simulink model "ofdm_txrx_supermimo_coded"  (Simulink 7.7)
settings: Solver=VariableStepDiscrete  SolverMode=SingleTasking  StartTime=0.0  StopTime=2*simOnly_simLength  RelTol=1e-3
PostLoadFcn:
  | ofdm_rx_supermimo_init
InitFcn:
  | ofdm_rx_supermimo_init
StartFcn:
  | ofdm_rx_supermimo_init
values from neighbouring files (symbols this model uses):
  ActionID_AFTransmit = 31    [ofdm_tx_supermimo_init.m]
  ActionID_Disabled = 0    [ofdm_tx_supermimo_init.m]
  ActionID_SetFlagA = 62    [ofdm_tx_supermimo_init.m]
  ActionID_SetFlagB = 61    [ofdm_tx_supermimo_init.m]
  BER_RAM_init_size = 2048    [ofdm_tx_supermimo_init.m]
  channel_h = [1 0; 1 0] * 0.3    [ofdm_tx_supermimo_init.m]
  CPLength = 16    [ofdm_tx_supermimo_init.m]
  longCorr_coef_i = [-4*real(LTS) 0]    [ofdm_rx_supermimo_init.m]
  longCorr_coef_q = [4*imag(LTS) 0]    [ofdm_rx_supermimo_init.m]
  max_CP_length = 16    [ofdm_tx_supermimo_init.m]
  max_num_baseRateSymbols = 31    [ofdm_tx_supermimo_init.m]
  max_num_subcarriers = 64    [ofdm_tx_supermimo_init.m]
  max_num_trainingSymbols = 15    [ofdm_tx_supermimo_init.m]
  max_numBytes = 16384    [ofdm_tx_supermimo_init.m]
  max_OFDM_symbols = 1023    [ofdm_tx_supermimo_init.m]
  modConstellation_qam16 = .75*[1 3 -1 -3]./3    [ofdm_tx_supermimo_init.m]
  modConstellation_qam64 = 0.875*[3 1 5 7 -3 -1 -5 -7]./7    [ofdm_tx_supermimo_init.m]
  modConstellation_qpsk = [1 -1]./sqrt(2)    [ofdm_tx_supermimo_init.m]
  numBaseRateSymbols = numBaseRateSymbols *2    [ofdm_tx_supermimo_init.m]
  numSubcarriers = 64    [ofdm_tx_supermimo_init.m]
  numTrainingSymbols = 2    [ofdm_tx_supermimo_init.m]
  pktTiming_count_payloadStart = pktTiming_count_lsStart + pktTiming_ls_num*pktTiming_ls_length    [ofdm_rx_supermimo_init.m]
  preamble = preamble_scale*[shortsyms_10 longsyms_2]    [ofdm_tx_supermimo_init.m]
  preamble_ROM = preamble_I_ROM + preamble_Q_ROM*2^16    [ofdm_tx_supermimo_init.m]
  RAM_init_size = max_numBytes    (= 16384)    [ofdm_tx_supermimo_init.m]
  RxDataScrambling_Seq = [40 198 78 63 82 173 102 245 48 111 172 115 147 230 216 93 72 65 62 2 205 242 122 90 128 83 105 97 73 10 5 252]    [ofdm_tx_supermimo_init.m]
  shortSymbol_time = shortSymbol_time(1:16).'    [ofdm_tx_supermimo_init.m]
  simOnly_numSamples = length(preamble)+( (numSubcarriers+CPLength)*(numTrainingSymbols + numBaseRateSymbols + numFullRateSymbols/2) )    [ofdm_tx_supermimo_init.m]
  train = [train train]    [ofdm_tx_supermimo_init.m]
  TxDataScrambling_Seq = [40 198 78 63 82 173 102 245 48 111 172 115 147 230 216 93 72 65 62 2 205 242 122 90 128 83 105 97 73 10 5 252]    [ofdm_tx_supermimo_init.m]
undefined symbols (not defined in the file or in its folder): AntA, AntB, BaseRate, Down, gcbh, numBuffs, numBytes, Up, xlBlockLoadCallback, xlGetNormalizedPeriod, xlipgetpartsetting
libraries: xbsIndex_r4 x5430
(part 34 of 49)

== system ofdm_txrx_supermimo_coded/OFDM Tx MIMO/Training_Data/FlexibleMod/PktBuffer_CRC1/Header Decoding1 ==
  TxByteAddr : Inport
  TxByte : Inport  Port=2
  TxByteAddrVin : Inport  Port=3
  Rst : Inport  Port=4
  4LSB : xbsIndex_r4/Slice  nbits=4  boolean_output=off  mode=Lower Bit Location + Width  bit1=0  base1=MSB of Input  bit0=0  base0=LSB of Input  dbl_ovrd=off  block_type=slice
      settings: infoedit="Extracts a given range of bits from each input sample and presents it at the output.  The output type is ordinarily unsigned with binary point at zero, but can be Boolean when the slice is one bit wide.<P><P>Hardware notes: In hardware this block costs nothing."  block_version=VER_STRING_GOES_HERE
  4MSB : xbsIndex_r4/Slice  nbits=4  boolean_output=off  mode=Upper Bit Location + Width  bit1=0  base1=MSB of Input  bit0=0  base0=LSB of Input  dbl_ovrd=off  block_type=slice
      settings: infoedit="Extracts a given range of bits from each input sample and presents it at the output.  The output type is ordinarily unsigned with binary point at zero, but can be Boolean when the slice is one bit wide.<P><P>Hardware notes: In hardware this block costs nothing."  block_version=VER_STRING_GOES_HERE
  8LSB : xbsIndex_r4/Slice  nbits=8  boolean_output=off  mode=Lower Bit Location + Width  bit1=0  base1=MSB of Input  bit0=0  base0=LSB of Input  dbl_ovrd=off  block_type=slice
      settings: infoedit="Extracts a given range of bits from each input sample and presents it at the output.  The output type is ordinarily unsigned with binary point at zero, but can be Boolean when the slice is one bit wide.<P><P>Hardware notes: In hardware this block costs nothing."  block_version=VER_STRING_GOES_HERE
  8LSB+8 : xbsIndex_r4/Slice  nbits=8  boolean_output=off  mode=Lower Bit Location + Width  bit1=0  base1=MSB of Input  bit0=8  base0=LSB of Input  dbl_ovrd=off  block_type=slice
      settings: infoedit="Extracts a given range of bits from each input sample and presents it at the output.  The output type is ordinarily unsigned with binary point at zero, but can be Boolean when the slice is one bit wide.<P><P>Hardware notes: In hardware this block costs nothing."  block_version=VER_STRING_GOES_HERE
  8MSB : xbsIndex_r4/Slice  nbits=8  boolean_output=off  mode=Upper Bit Location + Width  bit1=0  base1=MSB of Input  bit0=0  base0=LSB of Input  dbl_ovrd=off  block_type=slice
      settings: infoedit="Extracts a given range of bits from each input sample and presents it at the output.  The output type is ordinarily unsigned with binary point at zero, but can be Boolean when the slice is one bit wide.<P><P>Hardware notes: In hardware this block costs nothing."  block_version=VER_STRING_GOES_HERE
  Assert1 : xbsIndex_r4/Assert  assert_type=off  type_source=Explicitly  gui_display_data_type=Fixed-point  arith_type="Signed  (2's comp)"  n_bits=16  bin_pt=14  preci_type=Single  exp_width=8  frac_width=24  assert_rate=on  rate_source=Explicitly  period=2  output_port=on  block_type=assert
      settings: infoedit=Asserts a user-defined sample rate and/or type on a signal.<P><P>Hardware notes: In hardware this block costs nothing.  block_version=VER_STRING_GOES_HERE
  Assert2 : xbsIndex_r4/Assert  assert_type=off  type_source=Explicitly  gui_display_data_type=Fixed-point  arith_type="Signed  (2's comp)"  n_bits=16  bin_pt=14  preci_type=Single  exp_width=8  frac_width=24  assert_rate=on  rate_source=Explicitly  period=2  output_port=on  block_type=assert
      settings: infoedit=Asserts a user-defined sample rate and/or type on a signal.<P><P>Hardware notes: In hardware this block costs nothing.  block_version=VER_STRING_GOES_HERE
  Logical1 : xbsIndex_r4/Logical  logical_function=AND  inputs=2  en=off  latency=0  precision=Full  arith_type=Unsigned  n_bits=8  bin_pt=2  align_bp=on  dbl_ovrd=off  block_type=logical
      settings: xl_area=[1 0 0 1 0 0 0]  block_version=VER_STRING_GOES_HERE
  Logical2 : xbsIndex_r4/Logical  logical_function=AND  inputs=2  en=off  latency=0  precision=Full  arith_type=Unsigned  n_bits=8  bin_pt=2  align_bp=on  dbl_ovrd=off  block_type=logical
      settings: xl_area=[1 0 0 1 0 0 0]  block_version=VER_STRING_GOES_HERE
  Logical4 : xbsIndex_r4/Logical  logical_function=AND  inputs=2  en=off  latency=0  precision=Full  arith_type=Unsigned  n_bits=8  bin_pt=2  align_bp=on  dbl_ovrd=off  block_type=logical
      settings: xl_area=[1 0 0 1 0 0 0]  block_version=VER_STRING_GOES_HERE
  Register1 : xbsIndex_r4/Register  init=34  rst=on  en=on  dbl_ovrd=off  block_type=register
      settings: xl_area=[5 10 0 0 0 0 0]  block_version=VER_STRING_GOES_HERE
  Register2 : xbsIndex_r4/Register  init=0  rst=on  en=off  dbl_ovrd=off  block_type=register
      settings: xl_area=[5 10 0 0 0 0 0]  block_version=VER_STRING_GOES_HERE
  Relational1 : xbsIndex_r4/Relational  mode=a=b  en=off  latency=0  dbl_ovrd=off  block_type=relational
      settings: xl_area=[6 0 0 11 0 0 0]  block_version=VER_STRING_GOES_HERE
  Relational2 : xbsIndex_r4/Relational  mode=a=b  en=off  latency=0  dbl_ovrd=off  block_type=relational
      settings: xl_area=[6 0 0 11 0 0 0]  block_version=VER_STRING_GOES_HERE
  Relational3 : xbsIndex_r4/Relational  mode=a=b  en=off  latency=0  dbl_ovrd=off  block_type=relational
      settings: xl_area=[6 0 0 11 0 0 0]  block_version=VER_STRING_GOES_HERE
  numBytesUpdate : Subsystem
  numBytes : Outport
  modMask : Outport  Port=2
  wires:
  Relational3/1 -> Logical2/2
  8LSB+8/1 -> Relational3/2
  Relational2/1 -> Logical4/2
  8LSB/1 -> Relational2/2
  Rst/1 -> Register1/2, numBytesUpdate/1(rst), Register2/2
  Logical4/1 -> numBytesUpdate/3(lsb_EN)
  Logical2/1 -> numBytesUpdate/4(msb_EN)
  numBytesUpdate/1(numBytes) -> Assert1/1
  Assert1/1 -> numBytes/1
  [regRx_headerByteNums] -> 8LSB+8/1, 8LSB/1, 8MSB/1
  TxByteAddrVin/1 -> Logical4/1, Logical1/1, Logical2/1
  TxByte/1 -> numBytesUpdate/2(d), Register1/1
  Register2/1 -> Relational2/1, Relational3/1, Relational1/1
  Relational1/1 -> Logical1/2
  Logical1/1 -> Register1/3
  8MSB/1 -> Relational1/2
  Register1/1 -> Assert2/1
  Assert2/1 -> modMask/1, 4LSB/1, 4MSB/1
  4LSB/1 -> numBytesUpdate/6(modMask_AntB)
  4MSB/1 -> numBytesUpdate/5(modMask_AntA)
  TxByteAddr/1 -> Register2/1

== system ofdm_txrx_supermimo_coded/OFDM Tx MIMO/Training_Data/FlexibleMod/PktBuffer_CRC1/Packet Buffer ==
  note: For real-time BER testing, the Rx block uses this logic
      | to access the packet buffer. In hardware, Tx/Rx is half-duplex,
      | so sharing the logic is no problem. In simulation, it's full-duplex
      | (loopback, really), so some muxing is required.
  note: Use a long (12-bit) LFSR for more random payloads
      | Delay the Mux select by 1 cycle to line up with the latency
      | of the actual packet buffer (don't want to randomize the last
      | byte of the header!)
      | Polynomial set to dec2hex(2^11+2^8+2^6+2^0),
      | the optimal for a 12-bit LFSR per xapp210.
  note: Increment the LFSR on each new byte address. This
      | logic will "miss" byte0, but since we don't record
      | headers for random payloads, it doesn't matter.
  note: Disable random payload generation for
      | automatically transmitted packets
      | (either via autoTwoTx or via
      | autoResponders)
  TxByteAddr : Inport
  1LSB : xbsIndex_r4/Slice  nbits=1  boolean_output=on  mode=Lower Bit Location + Width  bit1=0  base1=MSB of Input  bit0=0  base0=LSB of Input  dbl_ovrd=off  block_type=slice
      settings: infoedit="Extracts a given range of bits from each input sample and presents it at the output.  The output type is ordinarily unsigned with binary point at zero, but can be Boolean when the slice is one bit wide.<P><P>Hardware notes: In hardware this block costs nothing."  block_version=8.2.02
  8LSB : xbsIndex_r4/Slice  nbits=8  boolean_output=off  mode=Lower Bit Location + Width  bit1=0  base1=MSB of Input  bit0=0  base0=LSB of Input  dbl_ovrd=off  block_type=slice
      settings: infoedit="Extracts a given range of bits from each input sample and presents it at the output.  The output type is ordinarily unsigned with binary point at zero, but can be Boolean when the slice is one bit wide.<P><P>Hardware notes: In hardware this block costs nothing."  block_version=8.2.02
  AutoTx Header Translation : Subsystem
  Convert{,1..3} (4 alike) : xbsIndex_r4/Convert  gui_display_data_type=Fixed-point  arith_type=Boolean  n_bits=16  bin_pt=14  float_type=Single  exp_bits=8  fraction_bits=24  quantization=Truncate  overflow=Wrap  en=off  latency=0  dbl_ovrd=off  pipeline=off  block_type=convert
      settings: block_version=10.1.3
  Delay{,1,3..6} (6 alike) : xbsIndex_r4/Delay  rst=off  infoeditControl=Selection of Reset will increase slice count due to use of real FFs and instead of SRLs  en=off  latency=1  dbl_ovrd=off  reg_retiming=off  block_type=delay
  Down Sample : xbsIndex_r4/Down Sample  sample_ratio=2  sample_phase="Last Value of Frame  (most efficient)"  en=off  latency=1  dbl_ovrd=off  block_type=dsamp
      settings: block_version=8.2.02
  Down Sample1 : xbsIndex_r4/Down Sample  sample_ratio=2  sample_phase="Last Value of Frame  (most efficient)"  en=off  latency=1  dbl_ovrd=off  block_type=dsamp
      settings: block_version=8.2.02
  Inverter1 : xbsIndex_r4/Inverter  en=off  latency=0  dbl_ovrd=off  block_type=inv
  Inverter2 : xbsIndex_r4/Inverter  en=off  latency=0  dbl_ovrd=off  block_type=inv
  Inverter3 : xbsIndex_r4/Inverter  en=off  latency=0  dbl_ovrd=off  block_type=inv
  LFSR : xbsIndex_r4/LFSR  lfsr_type=Fibonacci  gate_type=XOR  n_bits=12  polynomial=dec2hex(2^11+2^8+2^6+2^0)  rst_value='1'  rst=off  en=on  reloadable_seed=off  input_type=off  output_type=on  arith_type=Unsigned  bin_pt=0  dbl_ovrd=off  explicit_period=off  period=1  block_type=lfsr
      settings: block_version=10.1.3
  Logical1 : xbsIndex_r4/Logical  logical_function=AND  inputs=4  en=off  latency=0  precision=Full  arith_type=Unsigned  n_bits=8  bin_pt=2  align_bp=on  dbl_ovrd=off  block_type=logical
      settings: xl_area=[0, 0, 0, 0, 0, 0, 0]  block_version=VER_STRING_GOES_HERE
  Logical2 : xbsIndex_r4/Logical  logical_function=XOR  inputs=2  en=off  latency=0  precision=Full  arith_type=Unsigned  n_bits=8  bin_pt=2  align_bp=on  dbl_ovrd=off  block_type=logical
      settings: xl_area=[0, 0, 0, 0, 0, 0, 0]  block_version=VER_STRING_GOES_HERE
  Logical3 : xbsIndex_r4/Logical  logical_function=AND  inputs=2  en=off  latency=0  precision=Full  arith_type=Unsigned  n_bits=8  bin_pt=2  align_bp=on  dbl_ovrd=off  block_type=logical
      settings: xl_area=[0, 0, 0, 0, 0, 0, 0]  block_version=VER_STRING_GOES_HERE
  Mux1 : xbsIndex_r4/Mux  inputs=2  en=off  latency=0  precision=Full  arith_type=Unsigned  n_bits=16  bin_pt=14  quantization=Truncate  overflow=Wrap  dbl_ovrd=off  block_type=mux
      settings: block_version=8.2.02
  Mux2 : xbsIndex_r4/Mux  inputs=2  en=off  latency=0  precision=Full  arith_type=Unsigned  n_bits=16  bin_pt=14  quantization=Truncate  overflow=Wrap  dbl_ovrd=off  block_type=mux
      settings: block_version=8.2.02
  Pkt Buf IF : Subsystem
  Simulation Multiplexer : xbsIndex_r4/Simulation Multiplexer  sim_sel=1  hw_sel=2  block_type=simmux
      settings: infoedit="Distinguish input subsystems as \"simulation only\" and \"generation only\".  The input specified For Simulation will be used during Simulink simulation.  The input specified For Generation will be used during code generation.  This block will typically be used on the output of a HW Co-Simulation, Black Box, or ModelSim subsystem.<P><P>Hardware Notes: This block costs nothing."  block_version=10.1.3
  Tx Pkt Buf : Scope  NumInputPorts=8
      settings: TimeRange=8000  YMin=0~0~0~0~0~0~0~0  YMax=80~80~200~300~1~1~80~1  SaveName=ScopeData16
  Up Sample7 : xbsIndex_r4/Up Sample  sample_ratio=2  copy_samples=on  en=off  latency=0  dbl_ovrd=off  block_type=usamp
      settings: infoedit="Up samples input data.  Inserted values  can be zeros or copies of the most recent input sample.<P><P>Hardware notes: No hardware is needed if inserted values are copies of the input sample; otherwise, a mux and single bit flip-flop are used."  block_version=8.2.02
  done{1..7} (7 alike) : xbsIndex_r4/Gateway Out  inherit_from_input=off  hdl_port=off  timing_constraint=None  locs_specified=off  LOCs={}  UseAsDAC=off  DACChannel='1'  block_type=gatewayout
      settings: infoedit="Gateway out block.  Converts Xilinx fixed point inputs into ouputs of type Simulink integer, double, or fixed point.<P><P>Hardware notes:  In hardware these blocks become top level output ports or are discarded, depending on how they are configured."  xl_area=[0 0 0 0 1 0 0]  block_version=VER_STRING_GOES_HERE
  ByteOut : Outport
  tag scope: Tx_RandomPayloadByte=global, Tx_RandomPayloadByte_addr=global, Tx_RandomPayloadByte_valid=global
  wires:
  [regRx_FlexBERMode] -> Mux1/1
  [FlexBER_BRAM_Rx_ByteWrAddr] -> Mux1/3
  Mux1/1 -> Simulation Multiplexer/2
  Up Sample7/1 -> Mux1/2, Simulation Multiplexer/1
  AutoTx Header Translation/1(" ByteAddr") -> Up Sample7/1, done5/1
  Simulation Multiplexer/1 -> Pkt Buf IF/1(ByteAddr)
  Pkt Buf IF/1(ByteOut) -> Mux2/2, done3/1
  TxByteAddr/1 -> AutoTx Header Translation/1(ByteAddr), done4/1, 1LSB/1, Delay5/1
  AutoTx Header Translation/2(Header) -> Inverter2/1
  [regTx_RandomPayload] -> Convert1/1
  Logical1/1 -> Delay/1, Logical3/1
  Mux2/1 -> ByteOut/1
  Inverter2/1 -> Convert/1
  Convert/1 -> Logical1/1
  Convert1/1 -> Logical1/2
  LFSR/1 -> 8LSB/1
  8LSB/1 -> [Tx_RandomPayloadByte], Mux2/3, done6/1
  Delay/1 -> Mux2/1
  Delay1/1 -> [Tx_RandomPayloadByte_valid], done2/1
  1LSB/1 -> Logical2/1, Delay3/1
  Delay3/1 -> Logical2/2
  Logical3/1 -> Delay1/1, LFSR/1, done1/1
  Logical2/1 -> Delay4/1
  done4/1 -> Tx Pkt Buf/1
  done5/1 -> Tx Pkt Buf/2
  done3/1 -> Tx Pkt Buf/3
  done6/1 -> Tx Pkt Buf/4
  done1/1 -> Tx Pkt Buf/5
  Delay4/1 -> Logical3/2
  Delay5/1 -> Delay6/1
  Delay6/1 -> [Tx_RandomPayloadByte_addr], done7/1
  done2/1 -> Tx Pkt Buf/6
  done7/1 -> Tx Pkt Buf/7
  Inverter1/1 -> Convert2/1
  Convert2/1 -> Logical1/3
  [RxAutoReply_AutoTx] -> Down Sample/1
  [RxAutoReply_ExtTxEn] -> Down Sample1/1
  Inverter3/1 -> Convert3/1
  Convert3/1 -> Logical1/4
  Down Sample/1 -> Inverter1/1
  Down Sample1/1 -> Inverter3/1

== system ofdm_txrx_supermimo_coded/OFDM Tx MIMO/Training_Data/FlexibleMod/PktBuffer_CRC1/Scrambling ==
  Din : Inport
  Addr : Inport  Port=2
  5LSB : xbsIndex_r4/Slice  nbits=ceil(log2(length(TxDataScrambling_Seq)))  boolean_output=off  mode=Lower Bit Location + Width  bit1=0  base1=MSB of Input  bit0=0  base0=LSB of Input  dbl_ovrd=off  block_type=slice
      settings: infoedit="Extracts a given range of bits from each input sample and presents it at the output.  The output type is ordinarily unsigned with binary point at zero, but can be Boolean when the slice is one bit wide.<P><P>Hardware notes: In hardware this block costs nothing."  block_version=8.2.02
  Logical : xbsIndex_r4/Logical  logical_function=XOR  inputs=2  en=off  latency=0  precision=Full  arith_type=Unsigned  n_bits=16  bin_pt=0  align_bp=on  dbl_ovrd=off  block_type=logical
      settings: block_version=8.2.02
  ROM : xbsIndex_r4/ROM  depth=length(TxDataScrambling_Seq)  initVector=TxDataScrambling_Seq  distributed_mem=Distributed memory  rst=off  init_reg=0  en=off  latency=1  gui_display_data_type=Fixed-point  arith_type=Unsigned  n_bits=8  bin_pt=0  preci_type=Single  exp_width=8  frac_width=24  dbl_ovrd=off  optimize=Area  use_rpm=on  block_type=sprom
      settings: block_version=8.2.02
  Dout : Outport
  wires:
  ROM/1 -> Logical/2
  5LSB/1 -> ROM/1
  Logical/1 -> Dout/1
  Din/1 -> Logical/1
  Addr/1 -> 5LSB/1

== system ofdm_txrx_supermimo_coded/OFDM Tx MIMO/Training_Data/FlexibleMod/QAM/QPSK Map ==
  Bit : Inport
  Constant6 : xbsIndex_r4/Constant  const=modConstellation_qpsk(1)  gui_display_data_type=Fixed-point  arith_type=Signed (2's comp)  n_bits=modConstellation_prec  bin_pt=modConstellation_bp  preci_type=Single  exp_width=8  frac_width=24  explicit_period=off  period=1  equ=P=C  opselect=C  inp2=PCIN>>17  opr=+  inp1=P  carry=CIN  dbl_ovrd=off  block_type=constant
      settings: block_version=VER_STRING_GOES_HERE
  Constant7 : xbsIndex_r4/Constant  const=modConstellation_qpsk(2)  gui_display_data_type=Fixed-point  arith_type=Signed (2's comp)  n_bits=modConstellation_prec  bin_pt=modConstellation_bp  preci_type=Single  exp_width=8  frac_width=24  explicit_period=off  period=1  equ=P=C  opselect=C  inp2=PCIN>>17  opr=+  inp1=P  carry=CIN  dbl_ovrd=off  block_type=constant
      settings: block_version=VER_STRING_GOES_HERE
  Mux2 : xbsIndex_r4/Mux  inputs=2  en=off  latency=1  precision=Full  arith_type="Signed  (2's comp)"  n_bits=8  bin_pt=2  quantization=Truncate  overflow=Wrap  dbl_ovrd=off  block_type=mux
      settings: xl_area=[4 8 0 8 0 0 0]  block_version=VER_STRING_GOES_HERE
  Sym : Outport
  wires:
  Mux2/1 -> Sym/1
  Bit/1 -> Mux2/1
  Constant7/1 -> Mux2/3
  Constant6/1 -> Mux2/2

== system ofdm_txrx_supermimo_coded/OFDM Tx MIMO/Training_Data/FlexibleMod/QAM/QPSK Map1 ==
  Bit : Inport
  Constant6 : xbsIndex_r4/Constant  const=modConstellation_qpsk(1)  gui_display_data_type=Fixed-point  arith_type=Signed (2's comp)  n_bits=modConstellation_prec  bin_pt=modConstellation_bp  preci_type=Single  exp_width=8  frac_width=24  explicit_period=off  period=1  equ=P=C  opselect=C  inp2=PCIN>>17  opr=+  inp1=P  carry=CIN  dbl_ovrd=off  block_type=constant
      settings: block_version=VER_STRING_GOES_HERE
  Constant7 : xbsIndex_r4/Constant  const=modConstellation_qpsk(2)  gui_display_data_type=Fixed-point  arith_type=Signed (2's comp)  n_bits=modConstellation_prec  bin_pt=modConstellation_bp  preci_type=Single  exp_width=8  frac_width=24  explicit_period=off  period=1  equ=P=C  opselect=C  inp2=PCIN>>17  opr=+  inp1=P  carry=CIN  dbl_ovrd=off  block_type=constant
      settings: block_version=VER_STRING_GOES_HERE
  Mux2 : xbsIndex_r4/Mux  inputs=2  en=off  latency=1  precision=Full  arith_type="Signed  (2's comp)"  n_bits=8  bin_pt=2  quantization=Truncate  overflow=Wrap  dbl_ovrd=off  block_type=mux
      settings: xl_area=[4 8 0 8 0 0 0]  block_version=VER_STRING_GOES_HERE
  Sym : Outport
  wires:
  Constant6/1 -> Mux2/2
  Constant7/1 -> Mux2/3
  Bit/1 -> Mux2/1
  Mux2/1 -> Sym/1

== system ofdm_txrx_supermimo_coded/OFDM Tx MIMO/Training_Data/FlexibleMod/control/Concat1 ==
  in0 : Inport
  in1 : Inport  Port=2
  Concat1 : xbsIndex_r4/Concat  num_inputs=2  dbl_ovrd=off  block_type=concat
      settings: block_version=VER_STRING_GOES_HERE
  out0 : Outport
  wires:
  in0/1 -> Concat1/1
  in1/1 -> Concat1/2
  Concat1/1 -> out0/1

== system ofdm_txrx_supermimo_coded/OFDM Tx MIMO/Training_Data/FlexibleMod/control/Tx Modulation ==
  note: In Alamouti mode, we need two IFFT frames of data,
      | followed by two empty frames. The oscillator here does this
      | by forcing mod=0 for empty frames. The oscillator is reset
      | with each new packet (by posedge(baseRate)).
      | In SISO/Multiplexing, all of this is unnecessary.
  BaseRate : Inport
  Ant Sel : Inport  Port=2
  IFFT Index : Inport  Port=3
  FullRateMasks : Inport  Port=4
  4LSB : xbsIndex_r4/Slice  nbits=4  boolean_output=off  mode=Lower Bit Location + Width  bit1=0  base1=MSB of Input  bit0=0  base0=LSB of Input  dbl_ovrd=off  block_type=slice
      settings: infoedit="Extracts a given range of bits from each input sample and presents it at the output.  The output type is ordinarily unsigned with binary point at zero, but can be Boolean when the slice is one bit wide.<P><P>Hardware notes: In hardware this block costs nothing."  block_version=VER_STRING_GOES_HERE
  4MSB : xbsIndex_r4/Slice  nbits=4  boolean_output=off  mode=Upper Bit Location + Width  bit1=0  base1=MSB of Input  bit0=0  base0=LSB of Input  dbl_ovrd=off  block_type=slice
      settings: infoedit="Extracts a given range of bits from each input sample and presents it at the output.  The output type is ordinarily unsigned with binary point at zero, but can be Boolean when the slice is one bit wide.<P><P>Hardware notes: In hardware this block costs nothing."  block_version=VER_STRING_GOES_HERE
  AntB Mod Logic : Subsystem
  Concat1 : xbsIndex_r4/Concat  num_inputs=4  dbl_ovrd=off  block_type=concat
      settings: block_version=VER_STRING_GOES_HERE
  Concat2 : xbsIndex_r4/Concat  num_inputs=4  dbl_ovrd=off  block_type=concat
      settings: block_version=VER_STRING_GOES_HERE
  Concat3 : xbsIndex_r4/Concat  num_inputs=3  dbl_ovrd=off  block_type=concat
      settings: block_version=VER_STRING_GOES_HERE
  Inverter1 : xbsIndex_r4/Inverter  en=off  latency=0  dbl_ovrd=off  block_type=inv
      settings: block_version=VER_STRING_GOES_HERE
  Logical : xbsIndex_r4/Logical  logical_function=AND  inputs=2  en=off  latency=0  precision=Full  arith_type=Unsigned  n_bits=16  bin_pt=0  align_bp=on  dbl_ovrd=off  block_type=logical
      settings: block_version=VER_STRING_GOES_HERE
  Logical1 : xbsIndex_r4/Logical  logical_function=OR  inputs=2  en=off  latency=0  precision=Full  arith_type=Unsigned  n_bits=8  bin_pt=2  align_bp=on  dbl_ovrd=off  block_type=logical
      settings: xl_area=[1 0 0 1 0 0 0]  block_version=VER_STRING_GOES_HERE
  Logical2 : xbsIndex_r4/Logical  logical_function=AND  inputs=2  en=off  latency=0  precision=Full  arith_type=Unsigned  n_bits=8  bin_pt=2  align_bp=on  dbl_ovrd=off  block_type=logical
      settings: xl_area=[1 0 0 1 0 0 0]  block_version=VER_STRING_GOES_HERE
  Logical3 : xbsIndex_r4/Logical  logical_function=AND  inputs=2  en=off  latency=0  precision=Full  arith_type=Unsigned  n_bits=8  bin_pt=2  align_bp=on  dbl_ovrd=off  block_type=logical
      settings: xl_area=[1 0 0 1 0 0 0]  block_version=VER_STRING_GOES_HERE
  Logical4 : xbsIndex_r4/Logical  logical_function=OR  inputs=3  en=off  latency=0  precision=Full  arith_type=Unsigned  n_bits=16  bin_pt=0  align_bp=on  dbl_ovrd=off  block_type=logical
      settings: block_version=VER_STRING_GOES_HERE
  Mux1 : xbsIndex_r4/Mux  inputs=2  en=off  latency=0  precision=Full  arith_type=Unsigned  n_bits=16  bin_pt=14  quantization=Truncate  overflow=Wrap  dbl_ovrd=off  block_type=mux
      settings: block_version=8.2.02
  Oscillator : Subsystem
  Shared Memory for Modulation Masks : Subsystem
  negedge : Subsystem
  posedge : Subsystem
  mod : Outport
  wires:
  BaseRate/1 -> Concat2/1, Concat2/2, Concat2/3, Concat2/4, Concat3/1, posedge/1(d), AntB Mod Logic/1(BaseRate)
  IFFT Index/1 -> Concat3/3
  [TxReg_SISO_Mode] -> Logical2/2
  Concat3/1 -> Shared Memory for Modulation Masks/1(Addr)
  Logical/1 -> mod/1
  FullRateMasks/1 -> 4LSB/1, 4MSB/1
  4LSB/1 -> Mux1/2
  4MSB/1 -> Mux1/3
  Mux1/1 -> Logical4/2
  Logical4/1 -> Logical/1
  [regRx_simpleDynRxModulationEn] -> Inverter1/1
  Inverter1/1 -> Concat1/1, Concat1/2, Concat1/3, Concat1/4
  Concat2/1 -> Logical4/3
  Ant Sel/1 -> Mux1/1, Logical2/1, negedge/1(d), AntB Mod Logic/2(AntSel)
  Concat1/1 -> Logical4/1
  Shared Memory for Modulation Masks/1(ModMask) -> Logical/2
  Logical2/1 -> Logical1/1
  Logical1/1 -> Shared Memory for Modulation Masks/2(Rst)
  negedge/1(q) -> Oscillator/2(en)
  Logical3/1 -> Logical1/2
  [TxReg_Alamouti_Mode] -> Logical3/1
  Oscillator/1(Q) -> Logical3/2
  AntB Mod Logic/1(AntB Mod En) -> Concat3/2
  posedge/1(q) -> Oscillator/1(rst)

== system ofdm_txrx_supermimo_coded/OFDM Tx MIMO/Training_Data/FlexibleMod/control/ending ==
  signal : Inport
  Delay2 : xbsIndex_r4/Delay  rst=off  infoeditControl=Selection of Reset will increase slice count due to use of real FFs and instead of SRLs  en=off  latency=1  dbl_ovrd=off  reg_retiming=off  block_type=delay
      settings: infoedit=Hardware notes: A delay line is a chain, each link of which is an SRL16 followed by a flip-flop. If register retiming is enabled, the delay line is a chain of flip-flops.  xl_area=[1 1 0 0 0 0 0]  block_version=VER_STRING_GOES_HERE
  Inverter3 : xbsIndex_r4/Inverter  en=off  latency=0  dbl_ovrd=off  block_type=inv
      settings: xl_area=[0 0 0 0 0 0 0]  block_version=VER_STRING_GOES_HERE
  Logical2 : xbsIndex_r4/Logical  logical_function=AND  inputs=2  en=off  latency=0  precision=Full  arith_type=Unsigned  n_bits=8  bin_pt=2  align_bp=on  dbl_ovrd=off  block_type=logical
      settings: xl_area=[1 0 0 1 0 0 0]  block_version=VER_STRING_GOES_HERE
  pulse : Outport
  wires:
  Delay2/1 -> Logical2/2
  Inverter3/1 -> Delay2/1
  signal/1 -> Logical2/1, Inverter3/1
  Logical2/1 -> pulse/1

== system ofdm_txrx_supermimo_coded/OFDM Tx MIMO/Training_Data/FlexibleMod/posedge1/Delay3 ==
  in0 : Inport
  Delay3 : xbsIndex_r4/Delay  rst=off  infoeditControl=Selection of Reset will increase slice count due to use of real FFs and instead of SRLs  en=off  latency=1  dbl_ovrd=off  reg_retiming=off  block_type=delay
      settings: infoedit=Hardware notes: A delay line is a chain, each link of which is an SRL16 followed by a flip-flop. If register retiming is enabled, the delay line is a chain of flip-flops.  xl_area=[1 1 0 0 0 0 0]  block_version=VER_STRING_GOES_HERE
  out0 : Outport
  wires:
  in0/1 -> Delay3/1
  Delay3/1 -> out0/1

== system ofdm_txrx_supermimo_coded/OFDM Tx MIMO/Training_Data/FlexibleMod/posedge1/Inverter2 ==
  in0 : Inport
  Inverter2 : xbsIndex_r4/Inverter  en=off  latency=0  dbl_ovrd=off  block_type=inv
      settings: xl_area=[0 0 0 0 0 0 0]  block_version=VER_STRING_GOES_HERE
  out0 : Outport
  wires:
  in0/1 -> Inverter2/1
  Inverter2/1 -> out0/1

== system ofdm_txrx_supermimo_coded/OFDM Tx MIMO/Training_Data/FlexibleMod/posedge1/Logical3 ==
  in0 : Inport
  in1 : Inport  Port=2
  Logical3 : xbsIndex_r4/Logical  logical_function=AND  inputs=2  en=off  latency=0  precision=Full  arith_type=Unsigned  n_bits=8  bin_pt=2  align_bp=on  dbl_ovrd=off  block_type=logical
      settings: xl_area=[1 0 0 1 0 0 0]  block_version=VER_STRING_GOES_HERE
  out0 : Outport
  wires:
  in0/1 -> Logical3/1
  in1/1 -> Logical3/2
  Logical3/1 -> out0/1

== system ofdm_txrx_supermimo_coded/OFDM Tx MIMO/Training_Data/FlexibleMod/posedge2/Delay3 ==
  in0 : Inport
  Delay3 : xbsIndex_r4/Delay  rst=off  infoeditControl=Selection of Reset will increase slice count due to use of real FFs and instead of SRLs  en=off  latency=1  dbl_ovrd=off  reg_retiming=off  block_type=delay
      settings: infoedit=Hardware notes: A delay line is a chain, each link of which is an SRL16 followed by a flip-flop. If register retiming is enabled, the delay line is a chain of flip-flops.  xl_area=[1 1 0 0 0 0 0]  block_version=VER_STRING_GOES_HERE
  out0 : Outport
  wires:
  in0/1 -> Delay3/1
  Delay3/1 -> out0/1

== system ofdm_txrx_supermimo_coded/OFDM Tx MIMO/Training_Data/FlexibleMod/posedge2/Inverter2 ==
  in0 : Inport
  Inverter2 : xbsIndex_r4/Inverter  en=off  latency=0  dbl_ovrd=off  block_type=inv
      settings: xl_area=[0 0 0 0 0 0 0]  block_version=VER_STRING_GOES_HERE
  out0 : Outport
  wires:
  in0/1 -> Inverter2/1
  Inverter2/1 -> out0/1

== system ofdm_txrx_supermimo_coded/OFDM Tx MIMO/Training_Data/FlexibleMod/posedge2/Logical3 ==
  in0 : Inport
  in1 : Inport  Port=2
  Logical3 : xbsIndex_r4/Logical  logical_function=AND  inputs=2  en=off  latency=0  precision=Full  arith_type=Unsigned  n_bits=8  bin_pt=2  align_bp=on  dbl_ovrd=off  block_type=logical
      settings: xl_area=[1 0 0 1 0 0 0]  block_version=VER_STRING_GOES_HERE
  out0 : Outport
  wires:
  in0/1 -> Logical3/1
  in1/1 -> Logical3/2
  Logical3/1 -> out0/1

== system ofdm_txrx_supermimo_coded/OFDM Tx MIMO/Training_Data/Training_Pilots/Scrambled_Pilot_Inserter1/Pilot Scrambling Sequence Gen ==
  rst : Inport
  en : Inport  Port=2
  Assert : xbsIndex_r4/Assert  assert_type=on  type_source=Explicitly  gui_display_data_type=Fixed-point  arith_type=Boolean  n_bits=16  bin_pt=14  preci_type=Single  exp_width=8  frac_width=24  assert_rate=on  rate_source=Explicitly  period=2  output_port=on  block_type=assert
      settings: infoedit=Asserts a user-defined sample rate and/or type on a signal.<P><P>Hardware notes: In hardware this block costs nothing.  block_version=VER_STRING_GOES_HERE
  Assert1 : xbsIndex_r4/Assert  assert_type=on  type_source=Explicitly  gui_display_data_type=Fixed-point  arith_type=Boolean  n_bits=16  bin_pt=14  preci_type=Single  exp_width=8  frac_width=24  assert_rate=on  rate_source=Explicitly  period=2  output_port=on  block_type=assert
      settings: infoedit=Asserts a user-defined sample rate and/or type on a signal.<P><P>Hardware notes: In hardware this block costs nothing.  block_version=VER_STRING_GOES_HERE
  Convert : xbsIndex_r4/Convert  gui_display_data_type=Fixed-point  arith_type=Boolean  n_bits=1  bin_pt=0  float_type=Single  exp_bits=8  fraction_bits=24  quantization=Truncate  overflow=Wrap  en=off  latency=0  dbl_ovrd=off  pipeline=off  block_type=convert
      settings: xl_area=[0, 0, 0, 0, 0, 0, 0]  block_version=VER_STRING_GOES_HERE
  Logical : xbsIndex_r4/Logical  logical_function=XOR  inputs=2  en=off  latency=0  precision=Full  arith_type=Unsigned  n_bits=8  bin_pt=2  align_bp=on  dbl_ovrd=off  block_type=logical
      settings: xl_area=[0, 0, 0, 0, 0, 0, 0]  block_version=VER_STRING_GOES_HERE
  Logical1 : xbsIndex_r4/Logical  logical_function=AND  inputs=2  en=off  latency=0  precision=Full  arith_type=Unsigned  n_bits=8  bin_pt=2  align_bp=on  dbl_ovrd=off  block_type=logical
      settings: xl_area=[0, 0, 0, 0, 0, 0, 0]  block_version=VER_STRING_GOES_HERE
  Register{,1..6} (7 alike) : xbsIndex_r4/Register  init=1  rst=on  en=on  dbl_ovrd=off  block_type=register
      settings: xl_area=[0, 0, 0, 0, 0, 0, 0]  block_version=VER_STRING_GOES_HERE
  Q : Outport
  wires:
  rst/1 -> Register/2, Register1/2, Register2/2, Register3/2, Register4/2, Register5/2, Register6/2
  Register6/1 -> Assert/1
  Logical1/1 -> Register/3, Register1/3, Register2/3, Register3/3, Register4/3, Register5/3, Register6/3
  Logical/1 -> Register/1, Convert/1
  Register5/1 -> Register6/1
  Register4/1 -> Register5/1
  Register3/1 -> Register4/1, Assert1/1
  Register2/1 -> Register3/1
  Register1/1 -> Register2/1
  Register/1 -> Register1/1
  Convert/1 -> Q/1
  Assert/1 -> Logical/1
  Assert1/1 -> Logical/2
  en/1 -> Logical1/1
  [TxReg_PilotScrambling] -> Logical1/2

== system ofdm_txrx_supermimo_coded/OFDM Tx MIMO/Training_Data/Training_Pilots/Scrambled_Pilot_Inserter1/Pilot Values ==
  16LSB : xbsIndex_r4/Slice  nbits=16  boolean_output=off  mode=Lower Bit Location + Width  bit1=0  base1=MSB of Input  bit0=0  base0=LSB of Input  dbl_ovrd=off  block_type=slice
      settings: infoedit="Extracts a given range of bits from each input sample and presents it at the output.  The output type is ordinarily unsigned with binary point at zero, but can be Boolean when the slice is one bit wide.<P><P>Hardware notes: In hardware this block costs nothing."  block_version=VER_STRING_GOES_HERE
  16MSB : xbsIndex_r4/Slice  nbits=16  boolean_output=off  mode=Upper Bit Location + Width  bit1=0  base1=MSB of Input  bit0=0  base0=LSB of Input  dbl_ovrd=off  block_type=slice
      settings: infoedit="Extracts a given range of bits from each input sample and presents it at the output.  The output type is ordinarily unsigned with binary point at zero, but can be Boolean when the slice is one bit wide.<P><P>Hardware notes: In hardware this block costs nothing."  block_version=VER_STRING_GOES_HERE
  Down Sample1 : xbsIndex_r4/Down Sample  sample_ratio=2  sample_phase="Last Value of Frame  (most efficient)"  en=off  latency=1  dbl_ovrd=off  block_type=dsamp
      settings: block_version=VER_STRING_GOES_HERE
  Down Sample3 : xbsIndex_r4/Down Sample  sample_ratio=2  sample_phase="Last Value of Frame  (most efficient)"  en=off  latency=1  dbl_ovrd=off  block_type=dsamp
      settings: block_version=VER_STRING_GOES_HERE
  Reinterpret : xbsIndex_r4/Reinterpret  force_arith_type=on  arith_type="Signed  (2's comp)"  force_bin_pt=on  bin_pt=15  block_type=cast
      settings: infoedit="Changes type of samples without altering their binary representation.<P><P>Hardware notes: In hardware this block costs nothing.<P><P>Example:  Suppose the input is 6 bits wide, signed, with 2 fractional bits, and the output is forced to unsigned with 0 fractional bits.  Then an input of -2.0 (1110.00 in binary 2's complement) becomes an output of 56 (111000 in binary)."  block_version=VER_STRING_GOES_HERE
  Reinterpret2 : xbsIndex_r4/Reinterpret  force_arith_type=on  arith_type="Signed  (2's comp)"  force_bin_pt=on  bin_pt=15  block_type=cast
      settings: infoedit="Changes type of samples without altering their binary representation.<P><P>Hardware notes: In hardware this block costs nothing.<P><P>Example:  Suppose the input is 6 bits wide, signed, with 2 fractional bits, and the output is forced to unsigned with 0 fractional bits.  Then an input of -2.0 (1110.00 in binary 2's complement) becomes an output of 56 (111000 in binary)."  block_version=VER_STRING_GOES_HERE
  val1 : Outport
  val2 : Outport  Port=2
  wires:
  [regTx_PilotToneValues] -> 16LSB/1, 16MSB/1
  16LSB/1 -> Reinterpret/1
  16MSB/1 -> Reinterpret2/1
  Reinterpret/1 -> Down Sample3/1
  Reinterpret2/1 -> Down Sample1/1
  Down Sample3/1 -> val2/1
  Down Sample1/1 -> val1/1

== system ofdm_txrx_supermimo_coded/OFDM Rx MIMO/AF Buffers/AF Buffers/Read Control/AF Preamble Counter/PreambleEn ==
  R : Inport
  S : Inport  Port=2
  Constant4 : xbsIndex_r4/Constant  const=1  gui_display_data_type=Fixed-point  arith_type=Boolean  n_bits=1  bin_pt=0  preci_type=Single  exp_width=8  frac_width=24  explicit_period=off  period=2  equ=P=C  opselect=C  inp2=PCIN>>17  opr=+  inp1=P  carry=CIN  dbl_ovrd=off  block_type=constant
      settings: block_version=VER_STRING_GOES_HERE
  Convert : xbsIndex_r4/Convert  gui_display_data_type=Fixed-point  arith_type=Boolean  n_bits=16  bin_pt=14  float_type=Single  exp_bits=8  fraction_bits=24  quantization=Truncate  overflow=Wrap  en=off  latency=0  dbl_ovrd=off  pipeline=off  block_type=convert
      settings: block_version=10.1.3
  Convert1 : xbsIndex_r4/Convert  gui_display_data_type=Fixed-point  arith_type=Boolean  n_bits=16  bin_pt=14  float_type=Single  exp_bits=8  fraction_bits=24  quantization=Truncate  overflow=Wrap  en=off  latency=0  dbl_ovrd=off  pipeline=off  block_type=convert
      settings: block_version=10.1.3
  Register1 : xbsIndex_r4/Register  init=0  rst=on  en=on  dbl_ovrd=off  block_type=register
      settings: xl_area=[5 10 0 0 0 0 0]  block_version=VER_STRING_GOES_HERE
  Q : Outport
  wires:
  Convert1/1 -> Register1/3
  Convert/1 -> Register1/2
  S/1 -> Convert1/1
  Register1/1 -> Q/1
  R/1 -> Convert/1
  Constant4/1 -> Register1/1
